simulink model "TosAeb"  (R2021a)
settings: Solver=FixedStepDiscrete  StartTime=0.0  StopTime=inf  FixedStep=0.01  RelTol=1e-3
undefined symbols (not defined in the file or in its folder): ACCModSts, AccSts1, AEB_ObjectMovingOrientation, AEB_ObjectMovingState, CN_BTCActive, CN_CurrentGearR, CN_CurrentGearR_DHT, CN_PTCActive, CN_VDCActive, CN_VSC_EspOff, CN_VSC_FCW_AEB_Error, CN_VSC_FCW_AEB_NoDispay, CN_VSC_FCW_AEB_PerfmDgdtn, CN_VSC_FCW_AEB_TempUnAvlbl, CN_VSC_VehStandstill, CNaccelAvoidMax, CNaccelAvoidMin, CNbrakePedalPress, CNcomfortStrLeftAccelMax, CNcomfortStrLeftAccelMin, CNcomfortStrRightAccelMax, CNcomfortStrRightAccelMin, CNDrvDoorOpen, CNDrvModeEco, CNDrvModeNorm, CNDrvModeOther, CNDrvModeSport, CNDrvSBRreminder, CNNull, CNobjInvalidID, CNPassDoorOpen, CNPassSBRreminder, CNPEBSAccelNegInf, CNPEBSAccelNull, CNPEBSTimeInf, CNPEBSTimeNull, CNSTOPMAX, CNSTOPNULL, CNsubjWidth, CNSwtOFF, CNSwtON, CNTTCMAX, CNTTCNULL, CNVehConfigAEBCycNone, CNVehConfigAEBNone, CNVehConfigAEBPedNone, CNVehConfigFCWNone, CNVehConfigJANone, CollisionSeverity, FALSE, FCWSnvty, JACycObjCalcInfoDefaultParams, JAPedObjCalcInfoDefaultParams, JAVehObjCalcInfoDefaultParams, latCycObjCalcInfoDefaultParams, latPedObjCalcInfoDefaultParams, longObjCalcInfoDefaultParams, ModSts, movingStatus, ObjectType ... (+1112)
libraries: simulink x3031, AEBSFunctionLib x50, Ta_Library x33, AEBSStateMachineLib x23, Mylibrary x12, simulink_extras x7, ObjSelect_lib x3, dspstat3 x2
(part 40 of 102)

== system TosAeb/FcwAebJa_Step_sys/ALG_AEBSystem/ALG_AEBController/AEB_FuncCondCalcApp/JaVehFunctionCondCalc/AEBJAVehPrefillSuppAborCond/AEBJAVehPrefillSuppCondCalc/AEBJAVehPrefillSuppObjCondCalcu ==
  note: add Active
  ObjectMovingState : Inport
  ObjectMovingOrientation : Inport  Port=2
  ObjectID : Inport  Port=3
  ObjectExistProb : Inport  Port=4
  ObjectIsObstacleProb : Inport  Port=5
  ObjectTypeCameraConfirmed : Inport  Port=6
  dy : Inport  Port=7
  vxAbsObj : Inport  Port=8
  vyRelCorr : Inport  Port=9
  ObjFusQuality : Inport  Port=10
  Compare To Constant1 : simulink/Logic and Bit Operations/Compare To Constant  relop=>  const=P_AEB_Func_JaVehPrefillObjVelSupThresMin  OutDataTypeStr=boolean
  Compare To Constant11 : simulink/Logic and Bit Operations/Compare To Constant  relop===  const=TRUE  OutDataTypeStr=boolean
  Compare To Constant14 : simulink/Logic and Bit Operations/Compare To Constant  relop===  const=AEB_ObjectMovingState.Moving  OutDataTypeStr=boolean
  Compare To Constant15 : simulink/Logic and Bit Operations/Compare To Constant  relop=~=  const=CNobjInvalidID  OutDataTypeStr=boolean
  Compare To Constant16 : simulink/Logic and Bit Operations/Compare To Constant  relop=>  const=P_AEB_Func_JaVehPrefillObjObstacleProbSupThres  OutDataTypeStr=boolean
  Compare To Constant17 : simulink/Logic and Bit Operations/Compare To Constant  relop=~=  const=CNobjInvalidID  OutDataTypeStr=boolean
  Compare To Constant18 : simulink/Logic and Bit Operations/Compare To Constant  relop===  const=TRUE  OutDataTypeStr=boolean
  Compare To Constant2 : simulink/Logic and Bit Operations/Compare To Constant  relop=<  const=P_AEB_Func_JaVehPrefillObjVelSupThresMax  OutDataTypeStr=boolean
  Compare To Constant3 : simulink/Logic and Bit Operations/Compare To Constant  relop=>  const=P_AEB_Func_JaVehPrefillObjDySupThresMin  OutDataTypeStr=boolean
  Compare To Constant4 : simulink/Logic and Bit Operations/Compare To Constant  relop===  const=AEB_ObjectMovingOrientation.InvDir  OutDataTypeStr=boolean
  Compare To Constant5 : simulink/Logic and Bit Operations/Compare To Constant  relop=<  const=P_AEB_Func_JaVehPrefillObjDySupThresMax  OutDataTypeStr=boolean
  Logical Operator{1..2,6..7} (4 alike) : Logic  AllPortsSameDT=off  OutDataTypeStr=boolean
  Logical Operator4 : Logic  Inputs=8  AllPortsSameDT=off  OutDataTypeStr=boolean
  Math Function : Math  Operator=hypot  SignedPower=on  SaturateOnIntegerOverflow=on
  Product1 : Product
  Relational Operator2 : RelationalOperator  Operator===  OutDataTypeStr=boolean  RndMeth=Simplest
  Unit Delay1 : UnitDelay  SampleTime=-1  HasFrameUpgradeWarning=on
  AEBJAVehPrefillSuppObjActiveCondFulfilled : Outport
  wires:
  Compare To Constant11/1 -> Logical Operator4/8  "ObjFusQuality"
  ObjFusQuality/1 -> Compare To Constant11/1
  Logical Operator2/1 -> Logical Operator4/6  "objVelocityCondFulfilled"
  Compare To Constant5/1 -> Logical Operator2/2
  Compare To Constant3/1 -> Logical Operator2/1
  dy/1 -> Compare To Constant5/1, Compare To Constant3/1
  Math Function/1 -> Compare To Constant1/1, Compare To Constant2/1
  Logical Operator4/1 -> AEBJAVehPrefillSuppObjActiveCondFulfilled/1
  Logical Operator1/1 -> Logical Operator4/7  "objVelocityCondFulfilled"
  Compare To Constant2/1 -> Logical Operator1/2
  Compare To Constant1/1 -> Logical Operator1/1
  vyRelCorr/1 -> Math Function/2
  vxAbsObj/1 -> Math Function/1
  Compare To Constant18/1 -> Logical Operator4/5  "objFusionCondFulfilled"
  ObjectTypeCameraConfirmed/1 -> Compare To Constant18/1
  Logical Operator7/1 -> Logical Operator4/4  "objQualityCondFulfilled"
  Compare To Constant17/1 -> Logical Operator7/1
  Compare To Constant16/1 -> Logical Operator7/2
  ObjectIsObstacleProb/1 -> Product1/2
  Product1/1 -> Compare To Constant16/1
  ObjectExistProb/1 -> Product1/1
  ObjectID/1 -> Compare To Constant15/1, Relational Operator2/1, Unit Delay1/1, Compare To Constant17/1
  Unit Delay1/1 -> Relational Operator2/2
  Relational Operator2/1 -> Logical Operator6/2
  Logical Operator6/1 -> Logical Operator4/3  "objIDCondFulfilled"
  Compare To Constant15/1 -> Logical Operator6/1
  Compare To Constant4/1 -> Logical Operator4/2  "objMovingOrientationCondFulfilled"
  ObjectMovingOrientation/1 -> Compare To Constant4/1
  Compare To Constant14/1 -> Logical Operator4/1  "objMovingStateCondFulfilled"
  ObjectMovingState/1 -> Compare To Constant14/1

== system TosAeb/FcwAebJa_Step_sys/ALG_AEBSystem/ALG_AEBController/AEB_FuncCondCalcApp/JaVehFunctionCondCalc/AEBJAVehPrefillSuppAborCond/AEBJAVehPrefillSuppCondCalc/AEBJAVehPrefillSuppSituationCondCalc ==
  initialLatDist : Inport
  restLatDist2Collision : Inport  Port=2
  tPredictTime : Inport  Port=3
  TTB : Inport  Port=4
  ObjColliFlg : Inport  Port=5
  Add : Sum  Inputs=2
  Add1 : Sum  Inputs=2
  Add2 : Sum  Inputs=2
  Compare To Constant : simulink/Logic and Bit Operations/Compare To Constant  relop===  const=TRUE  OutDataTypeStr=boolean
  Constant : Constant  Value=P_Global_VehicleWidthMin_m
  Constant1 : Constant  Value=P_AEB_Func_JaVehPrefillObjWidth
  Constant2 : Constant  Value=P_AEB_Func_JaVehPrefillBrkDistSecure
  Constant3 : Constant  Value=P_AEB_Func_JaVehAEBPrefillTtcTimeOffset
  Gain : Gain  Gain=0.5
  Gain1 : Gain  Gain=0.5
  Logical Operator : Logic  AllPortsSameDT=off  OutDataTypeStr=boolean
  Logical Operator1 : Logic  AllPortsSameDT=off  OutDataTypeStr=boolean
  Relational Operator : RelationalOperator  Operator=>  OutDataTypeStr=boolean  RndMeth=Simplest
  Relational Operator1 : RelationalOperator  Operator=<  OutDataTypeStr=boolean  RndMeth=Simplest
  Relational Operator2 : RelationalOperator  Operator=<=  OutDataTypeStr=boolean  RndMeth=Simplest
  Terminator : Terminator
  AEBJAVehPrefillActiveSituationCondFulfilled : Outport
  wires:
  restLatDist2Collision/1 -> Relational Operator2/1
  Relational Operator2/1 -> Logical Operator/2
  Relational Operator/1 -> Logical Operator/1
  Add/1 -> Add2/1
  Constant1/1 -> Gain1/1
  initialLatDist/1 -> Relational Operator/1
  Constant/1 -> Gain/1
  Gain/1 -> Add/1
  Gain1/1 -> Add/2
  Constant2/1 -> Add2/2
  Logical Operator/1 -> Terminator/1
  Add2/1 -> Relational Operator2/2, Relational Operator/2
  TTB/1 -> Add1/1
  tPredictTime/1 -> Relational Operator1/1
  Compare To Constant/1 -> Logical Operator1/2
  ObjColliFlg/1 -> Compare To Constant/1
  Add1/1 -> Relational Operator1/2
  Constant3/1 -> Add1/2
  Logical Operator1/1 -> AEBJAVehPrefillActiveSituationCondFulfilled/1
  Relational Operator1/1 -> Logical Operator1/1

== system TosAeb/FcwAebJa_Step_sys/ALG_AEBSystem/ALG_AEBController/AEB_FuncCondCalcApp/JaVehFunctionCondCalc/AEBJAVehPrefillSuppAborCond/AEBJAVehPrefillSuppCondCalc/AEBJAVehPrefillSuppSubjCondCalcu ==
  note: 2020.06.08 change or to and
  note: 2020.06.08 change and to or
  subjVel : Inport
  VehConfigIbooster : Inport  Port=2
  ESP_MasterCylindBrakePress : Inport  Port=3
  BrakePedalStatus_G : Inport  Port=4
  IB_BrkPedalAppld : Inport  Port=5
  AccrPedlPosnDiagc : Inport  Port=6
  SteerWheelAngle_Deg : Inport  Port=7
  LeftTurnSWSts : Inport  Port=8
  subjLatAccel : Inport  Port=9
  VSC_JAVehPrefillSuppCond : Inport  Port=10
  1-D Lookup Table : Lookup_n-D  NumberOfTableDimensions=1  BreakpointsForDimension1=P_AEB_Func_JaVehPrefillSubjVelStrWheelAngleMinThresX  BeginIndexSearchUsingPreviousIndexResult=on  Table=P_AEB_Func_JaVehPrefillSubjVelStrWheelAngleMinThresY  InternalRulePriority=Speed  RndMeth=Simplest  InputSameDT=on
  1-D Lookup Table1 : Lookup_n-D  NumberOfTableDimensions=1  BreakpointsForDimension1=P_AEB_Func_JaVehPrefillSubjVelStrWheelAngleMaxThresX  BeginIndexSearchUsingPreviousIndexResult=on  Table=P_AEB_Func_JaVehPrefillSubjVelStrWheelAngleMaxThresY  InternalRulePriority=Speed  RndMeth=Simplest  InputSameDT=on
  Abs : Abs
  Abs1 : Abs
  Compare To Constant2 : simulink/Logic and Bit Operations/Compare To Constant  relop===  const=FALSE  OutDataTypeStr=boolean
  Compare To Constant3 : simulink/Logic and Bit Operations/Compare To Constant  relop=<  const=P_AEB_Func_JaVehPrefillSubjLatAccelThres  OutDataTypeStr=boolean
  Compare To Constant4 : simulink/Logic and Bit Operations/Compare To Constant  relop===  const=FALSE  OutDataTypeStr=boolean
  Compare To Constant6 : simulink/Logic and Bit Operations/Compare To Constant  relop=>  const=P_AEB_Func_JaVehPrefillGasPedalSupThres  OutDataTypeStr=boolean
  Compare To Constant7 : simulink/Logic and Bit Operations/Compare To Constant  relop===  const=TRUE  OutDataTypeStr=boolean
  Compare To Constant8 : simulink/Logic and Bit Operations/Compare To Constant  relop=>=  const=P_AEB_Func_JaVehPrefillSubjVelMin  OutDataTypeStr=boolean
  Compare To Constant9 : simulink/Logic and Bit Operations/Compare To Constant  relop=<=  const=P_AEB_Func_JaVehPrefillSubjVelMax  OutDataTypeStr=boolean
  Constant : Constant  Value=P_AEB_Func_JaVehPrefillAccrCondAborDelayTime
  Constant1 : Constant  Value=Ts
  Constant2 : Constant  Value=P_AEB_Func_JaVehPrefillTurnSwtStsConfig
  Constant3 : Constant  Value=TRUE
  Logical Operator : Logic  AllPortsSameDT=off  OutDataTypeStr=boolean
  Logical Operator1 : Logic  Operator=OR  AllPortsSameDT=off  OutDataTypeStr=boolean
  Logical Operator4 : Logic  AllPortsSameDT=off  OutDataTypeStr=boolean
  Logical Operator5 : Logic  Inputs=6  AllPortsSameDT=off  OutDataTypeStr=boolean
  Relational Operator : RelationalOperator  Operator=>  OutDataTypeStr=boolean  RndMeth=Simplest
  Relational Operator1 : RelationalOperator  Operator=<  OutDataTypeStr=boolean  RndMeth=Simplest
  StateDelayAbortion : AEBSFunctionLib/StateDelayAbortion  SystemSampleTime=-1  VariantActivationTime=update diagram  Latency=0  AutoFrameSizeCalculation=off
  Switch : Switch  Criteria=u2 ~= 0
  brakeReleventSuppCond : Subsystem
  AEBJAVehPrefillVehActiveCondFulfilled : Outport
  wires:
  ESP_MasterCylindBrakePress/1 -> brakeReleventSuppCond/3(ESP_MasterCylindBrakePress)
  BrakePedalStatus_G/1 -> brakeReleventSuppCond/2(BrakePedalStatus_G)
  VehConfigIbooster/1 -> brakeReleventSuppCond/1(VehConfigIbooster)
  Compare To Constant7/1 -> Switch/1  "StrReleventActiveCondFulfilled"
  Constant2/1 -> Switch/2
  Constant3/1 -> Switch/3
  IB_BrkPedalAppld/1 -> brakeReleventSuppCond/4(IB_BrkPedalAppld)
  Compare To Constant2/1 -> Logical Operator5/2  "DrvReleventActiveCondFulfilled"
  Logical Operator1/1 -> Compare To Constant2/1
  Relational Operator1/1 -> Logical Operator4/2
  1-D Lookup Table1/1 -> Relational Operator1/2
  Compare To Constant4/1 -> Logical Operator5/6  "VSCReleventActiveCondFulfilled"
  VSC_JAVehPrefillSuppCond/1 -> Compare To Constant4/1
  Compare To Constant3/1 -> Logical Operator5/5  "LaterAccReleventActiveCondFulfilled"
  Abs1/1 -> Compare To Constant3/1
  subjLatAccel/1 -> Abs1/1
  1-D Lookup Table/1 -> Relational Operator/2
  [subjVel] -> 1-D Lookup Table/1, 1-D Lookup Table1/1
  Relational Operator/1 -> Logical Operator4/1
  Constant1/1 -> StateDelayAbortion/3
  Constant/1 -> StateDelayAbortion/2
  Compare To Constant6/1 -> StateDelayAbortion/1
  Logical Operator5/1 -> AEBJAVehPrefillVehActiveCondFulfilled/1  "AEBJAVehPrefillVehActiveCondFulfilled"
  subjVel/1 -> Compare To Constant8/1, [subjVel], Compare To Constant9/1
  Logical Operator/1 -> Logical Operator5/1  "VelocityReleventActiveCondFulfilled"
  Switch/1 -> Logical Operator5/4  "StrReleventActiveCondFulfilled"
  AccrPedlPosnDiagc/1 -> Compare To Constant6/1
  Compare To Constant9/1 -> Logical Operator/2
  brakeReleventSuppCond/1(brakeReleventSuppCond) -> Logical Operator1/1  "BrkReleventSuppCondFulfilled"
  Logical Operator4/1 -> Logical Operator5/3  "strReleventActiveCondFulfilled"
  SteerWheelAngle_Deg/1 -> Abs/1
  Abs/1 -> Relational Operator/1, Relational Operator1/1
  Compare To Constant8/1 -> Logical Operator/1
  LeftTurnSWSts/1 -> Compare To Constant7/1
  StateDelayAbortion/1 -> Logical Operator1/2  "accReleventSuppCondFulfilled"

== system TosAeb/FcwAebJa_Step_sys/ALG_AEBSystem/ALG_AEBController/AEB_FuncCondCalcApp/LongFunctionCondCalc/vehABASuppAborCondCalc/vehABAAborCondCalc/vehABAAborCondFinalJudge ==
  vehABAObjRelAborCondFulfilled : Inport
  vehABAVehRelAborCondFulfilled : Inport  Port=2
  vehABASituationRelAborCondFulfilled : Inport  Port=3
  Compare To Constant : simulink/Logic and Bit Operations/Compare To Constant  relop===  const=TRUE  OutDataTypeStr=boolean
  Compare To Constant1 : simulink/Logic and Bit Operations/Compare To Constant  relop===  const=TRUE  OutDataTypeStr=boolean
  Compare To Constant2 : simulink/Logic and Bit Operations/Compare To Constant  relop===  const=TRUE  OutDataTypeStr=boolean
  Logical Operator : Logic  Operator=OR  Inputs=3  AllPortsSameDT=off  OutDataTypeStr=boolean
  vehABAAborCondFulfilled : Outport
  wires:
  vehABAVehRelAborCondFulfilled/1 -> Compare To Constant1/1
  vehABAObjRelAborCondFulfilled/1 -> Compare To Constant/1
  vehABASituationRelAborCondFulfilled/1 -> Compare To Constant2/1
  Logical Operator/1 -> vehABAAborCondFulfilled/1
  Compare To Constant/1 -> Logical Operator/1
  Compare To Constant1/1 -> Logical Operator/3
  Compare To Constant2/1 -> Logical Operator/2

== system TosAeb/FcwAebJa_Step_sys/ALG_AEBSystem/ALG_AEBController/AEB_FuncCondCalcApp/LongFunctionCondCalc/vehABASuppAborCondCalc/vehABAAborCondCalc/vehABAObjRelAborCondCalc ==
  ObjectID : Inport
  Compare To Constant4 : simulink/Logic and Bit Operations/Compare To Constant  relop===  const=CNobjInvalidID  OutDataTypeStr=boolean
  Logical Operator1 : Logic  Operator=OR  AllPortsSameDT=off  OutDataTypeStr=boolean
  Relational Operator : RelationalOperator  Operator=~=  OutDataTypeStr=boolean  RndMeth=Simplest
  Unit Delay : UnitDelay  SampleTime=-1  HasFrameUpgradeWarning=on
  vehABAObjRelAborCondFulfilled : Outport
  wires:
  Logical Operator1/1 -> vehABAObjRelAborCondFulfilled/1  "ObjIDAborCondFulfilled"
  Compare To Constant4/1 -> Logical Operator1/1
  Relational Operator/1 -> Logical Operator1/2
  Unit Delay/1 -> Relational Operator/2
  ObjectID/1 -> Compare To Constant4/1, Relational Operator/1, Unit Delay/1

== system TosAeb/FcwAebJa_Step_sys/ALG_AEBSystem/ALG_AEBController/AEB_FuncCondCalcApp/LongFunctionCondCalc/vehABASuppAborCondCalc/vehABAAborCondCalc/vehABASituationRelAborCondCalc ==
  longCollisionSeverity : Inport
  Compare To Constant : simulink/Logic and Bit Operations/Compare To Constant  relop=~=  const=CollisionSeverity.Low  OutDataTypeStr=boolean
  Compare To Constant1 : simulink/Logic and Bit Operations/Compare To Constant  relop=~=  const=CollisionSeverity.Medium  OutDataTypeStr=boolean
  Compare To Constant2 : simulink/Logic and Bit Operations/Compare To Constant  relop=~=  const=CollisionSeverity.High  OutDataTypeStr=boolean
  Logical Operator : Logic  Inputs=3  AllPortsSameDT=off  OutDataTypeStr=boolean
  vehABASituationRelAborCondFulfilled : Outport
  wires:
  Logical Operator/1 -> vehABASituationRelAborCondFulfilled/1
  Compare To Constant/1 -> Logical Operator/1
  longCollisionSeverity/1 -> Compare To Constant/1, Compare To Constant2/1, Compare To Constant1/1
  Compare To Constant2/1 -> Logical Operator/3
  Compare To Constant1/1 -> Logical Operator/2

== system TosAeb/FcwAebJa_Step_sys/ALG_AEBSystem/ALG_AEBController/AEB_FuncCondCalcApp/LongFunctionCondCalc/vehABASuppAborCondCalc/vehABAAborCondCalc/vehABAVehRelAborCondCalc ==
  SteerWheelAngle : Inport
  SteerWheelSpd : Inport  Port=2
  AccrPedlPosnDiagc : Inport  Port=3
  vehStateCalcABAAborFlg : Inport  Port=4
  DriverAborCondJudge : Subsystem
  vehPreWarningVehRelActiveCondJudge : Subsystem
  vehABAVehRelAborCondFulfilled : Outport
  wires:
  AccrPedlPosnDiagc/1 -> DriverAborCondJudge/3(AccrPedlPosnDiagc)
  SteerWheelSpd/1 -> DriverAborCondJudge/2(SteerWheelSpd)
  SteerWheelAngle/1 -> DriverAborCondJudge/1(SteerWheelAngle)
  vehPreWarningVehRelActiveCondJudge/1(vehABAVehRelAborCondFulfilled) -> vehABAVehRelAborCondFulfilled/1
  vehStateCalcABAAborFlg/1 -> vehPreWarningVehRelActiveCondJudge/2(vehStateCalcABAAborFlg)
  DriverAborCondJudge/1(driverAborCondFlg) -> vehPreWarningVehRelActiveCondJudge/1(driverAborCondFlg)

== system TosAeb/FcwAebJa_Step_sys/ALG_AEBSystem/ALG_AEBController/AEB_FuncCondCalcApp/LongFunctionCondCalc/vehABASuppAborCondCalc/vehABASuppCondCalc/vehABAObjRelActiveCondCalc ==
  ObjectMovingState : Inport
  ObjectTypeCameraConfirmed : Inport  Port=2
  AEB_PedResp : Inport  Port=3
  ObjectType : Inport  Port=4
  subjCurvTraj : Inport  Port=5
  subjLatAccel : Inport  Port=6
  subjVel : Inport  Port=7
  vehConfigAEBCyc : Inport  Port=8
  vehConfigAEBPed : Inport  Port=9
  ObjectMovingOrientation : Inport  Port=10
  ObjectID : Inport  Port=11
  ObjectExistProb : Inport  Port=12
  ObjectIsObstacleProb : Inport  Port=13
  ObjFusQuality : Inport  Port=14
  Compare To Constant : simulink/Logic and Bit Operations/Compare To Constant  relop===  const=AEB_ObjectMovingState.Still  OutDataTypeStr=boolean
  Compare To Constant1 : simulink/Logic and Bit Operations/Compare To Constant  relop===  const=AEB_ObjectMovingState.Moving  OutDataTypeStr=boolean
  Compare To Constant10 : simulink/Logic and Bit Operations/Compare To Constant  relop===  const=TRUE  OutDataTypeStr=boolean
  Compare To Constant12 : simulink/Logic and Bit Operations/Compare To Constant  relop===  const=FALSE  OutDataTypeStr=boolean
  Compare To Constant2 : simulink/Logic and Bit Operations/Compare To Constant  relop===  const=AEB_ObjectMovingState.Stopped  OutDataTypeStr=boolean
  Compare To Constant3 : simulink/Logic and Bit Operations/Compare To Constant  relop===  const=AEB_ObjectMovingOrientation.SameDir  OutDataTypeStr=boolean
  Compare To Constant4 : simulink/Logic and Bit Operations/Compare To Constant  relop=~=  const=CNobjInvalidID  OutDataTypeStr=boolean
  Compare To Constant5 : simulink/Logic and Bit Operations/Compare To Constant  relop=>  const=P_AEB_Func_vehABAObjObstacleProbActivationThres  OutDataTypeStr=boolean
  Compare To Constant6 : simulink/Logic and Bit Operations/Compare To Constant  relop=~=  const=CNobjInvalidID  OutDataTypeStr=boolean
  Compare To Constant8 : simulink/Logic and Bit Operations/Compare To Constant  relop===  const=TRUE  OutDataTypeStr=boolean
  Constant3 : Constant  Value=P_AEB_Func_vehABASubjVelActivationThresForPedCyc
  Constant4 : Constant  Value=P_AEB_Func_vehABASubjCurvTrajActivationThresForPedCyc
  Constant5 : Constant  Value=P_AEB_Func_vehABASubjLatAccelActivationThresForPedCyc
  Logical Operator : Logic  Operator=OR  Inputs=3  AllPortsSameDT=off  OutDataTypeStr=boolean
  Logical Operator1 : Logic  AllPortsSameDT=off  OutDataTypeStr=boolean
  Logical Operator2 : Logic  AllPortsSameDT=off  OutDataTypeStr=boolean
  Logical Operator5 : Logic  Inputs=7  AllPortsSameDT=off  OutDataTypeStr=boolean
  PedCycObjCondSuppJudgeFlow : AEBSFunctionLib/PedCycObjCondSuppJudgeFlow  SystemSampleTime=-1  VariantActivationTime=update diagram  Latency=0  AutoFrameSizeCalculation=off
  Product : Product
  Relational Operator : RelationalOperator  Operator===  OutDataTypeStr=boolean  RndMeth=Simplest
  Unit Delay : UnitDelay  SampleTime=-1  HasFrameUpgradeWarning=on
  vehABAObjRelActiveCondFulfilled : Outport
  wires:
  Compare To Constant10/1 -> Logical Operator5/7  "ObjFusQuality"
  ObjFusQuality/1 -> Compare To Constant10/1
  Compare To Constant8/1 -> Logical Operator5/2  "ObjFusionInfoConfirm"
  Constant5/1 -> PedCycObjCondSuppJudgeFlow/11
  Constant4/1 -> PedCycObjCondSuppJudgeFlow/10
  Constant3/1 -> PedCycObjCondSuppJudgeFlow/9
  AEB_PedResp/1 -> PedCycObjCondSuppJudgeFlow/8
  vehConfigAEBPed/1 -> PedCycObjCondSuppJudgeFlow/7
  vehConfigAEBCyc/1 -> PedCycObjCondSuppJudgeFlow/6
  subjVel/1 -> PedCycObjCondSuppJudgeFlow/5
  subjLatAccel/1 -> PedCycObjCondSuppJudgeFlow/4
  subjCurvTraj/1 -> PedCycObjCondSuppJudgeFlow/3
  ObjectType/1 -> PedCycObjCondSuppJudgeFlow/2
  ObjectTypeCameraConfirmed/1 -> Compare To Constant8/1, PedCycObjCondSuppJudgeFlow/1
  Compare To Constant/1 -> Logical Operator/3
  Compare To Constant12/1 -> Logical Operator5/3  "pedCycReleventCondFulfilled"
  ObjectMovingState/1 -> Compare To Constant/1, Compare To Constant2/1, Compare To Constant1/1
  Compare To Constant2/1 -> Logical Operator/2
  Compare To Constant1/1 -> Logical Operator/1
  ObjectMovingOrientation/1 -> Compare To Constant3/1
  ObjectID/1 -> Compare To Constant6/1, Unit Delay/1, Relational Operator/1, Compare To Constant4/1
  Unit Delay/1 -> Relational Operator/2
  ObjectExistProb/1 -> Product/1
  ObjectIsObstacleProb/1 -> Product/2
  Compare To Constant6/1 -> Logical Operator2/1
  Compare To Constant5/1 -> Logical Operator2/2
  Product/1 -> Compare To Constant5/1
  Relational Operator/1 -> Logical Operator1/2
  Compare To Constant4/1 -> Logical Operator1/1
  Logical Operator5/1 -> vehABAObjRelActiveCondFulfilled/1
  Compare To Constant3/1 -> Logical Operator5/4  "objMovingOrientationCondFulfilled"
  Logical Operator2/1 -> Logical Operator5/6  "objQualityCondFulfilled"
  PedCycObjCondSuppJudgeFlow/1 -> Compare To Constant12/1  "pedCycObjCondSuppFlg"
  Logical Operator/1 -> Logical Operator5/1  "objMovingStateCondFulfilled"
  Logical Operator1/1 -> Logical Operator5/5  "objIDCondFulfilled"

== system TosAeb/FcwAebJa_Step_sys/ALG_AEBSystem/ALG_AEBController/AEB_FuncCondCalcApp/LongFunctionCondCalc/vehABASuppAborCondCalc/vehABASuppCondCalc/vehABASituationRelActiveCondCalc ==
  longCollisionSeverity : Inport
  overlapRatio : Inport  Port=2
  dxFrontBumper : Inport  Port=3
  probEscape : Inport  Port=4
  Compare To Constant : simulink/Logic and Bit Operations/Compare To Constant  relop===  const=CollisionSeverity.Low  OutDataTypeStr=boolean
  Compare To Constant1 : simulink/Logic and Bit Operations/Compare To Constant  relop===  const=CollisionSeverity.Medium  OutDataTypeStr=boolean
  Compare To Constant2 : simulink/Logic and Bit Operations/Compare To Constant  relop===  const=CollisionSeverity.High  OutDataTypeStr=boolean
  Compare To Constant3 : simulink/Logic and Bit Operations/Compare To Constant  relop=>  const=P_AEB_Func_vehABAEscapeActivationThres  OutDataTypeStr=boolean
  Compare To Constant5 : simulink/Logic and Bit Operations/Compare To Constant  relop===  const=FALSE  OutDataTypeStr=boolean
  Logical Operator : Logic  Operator=OR  Inputs=3  AllPortsSameDT=off  OutDataTypeStr=boolean
  Logical Operator1 : Logic  Inputs=3  AllPortsSameDT=off  OutDataTypeStr=boolean
  OverlapThesLookupTable : Lookup_n-D  NumberOfTableDimensions=1  BreakpointsForDimension1=P_AEB_Func_vehABAOverlapActivationThresX  BeginIndexSearchUsingPreviousIndexResult=on  Table=P_AEB_Func_vehABAOverlapActivationThresY  ExtrapMethod=Clip  InternalRulePriority=Speed  RndMeth=Simplest  InputSameDT=on
  Relational Operator : RelationalOperator  Operator=>  OutDataTypeStr=boolean  RndMeth=Simplest
  vehABASituationRelActiveCondFulfilled : Outport
  wires:
  Logical Operator1/1 -> vehABASituationRelActiveCondFulfilled/1
  Compare To Constant2/1 -> Logical Operator/3
  Compare To Constant/1 -> Logical Operator/1
  overlapRatio/1 -> Relational Operator/1
  Compare To Constant1/1 -> Logical Operator/2
  probEscape/1 -> Compare To Constant3/1
  longCollisionSeverity/1 -> Compare To Constant/1, Compare To Constant1/1, Compare To Constant2/1
  Compare To Constant3/1 -> Compare To Constant5/1  "probEscapeFulfilled"
  dxFrontBumper/1 -> OverlapThesLookupTable/1
  OverlapThesLookupTable/1 -> Relational Operator/2
  Relational Operator/1 -> Logical Operator1/2  "OverlapCondFulfilled"
  Logical Operator/1 -> Logical Operator1/1  "collisionLvlCondFulfilled"
  Compare To Constant5/1 -> Logical Operator1/3

== system TosAeb/FcwAebJa_Step_sys/ALG_AEBSystem/ALG_AEBController/AEB_FuncCondCalcApp/LongFunctionCondCalc/vehABASuppAborCondCalc/vehABASuppCondCalc/vehABASuppCondFinalJudge ==
  vehABAObjRelActiveCondFulfilled : Inport
  vehABAVehRelActiveCondFulfilled : Inport  Port=2
  vehABASituationRelActiveCondFulfilled : Inport  Port=3
  Compare To Constant : simulink/Logic and Bit Operations/Compare To Constant  relop===  const=FALSE  OutDataTypeStr=boolean
  Compare To Constant1 : simulink/Logic and Bit Operations/Compare To Constant  relop===  const=FALSE  OutDataTypeStr=boolean
  Compare To Constant2 : simulink/Logic and Bit Operations/Compare To Constant  relop===  const=FALSE  OutDataTypeStr=boolean
  Logical Operator : Logic  Operator=OR  Inputs=3  AllPortsSameDT=off  OutDataTypeStr=boolean
  vehABASuppCondFulfilled : Outport
  wires:
  vehABAVehRelActiveCondFulfilled/1 -> Compare To Constant1/1
  vehABAObjRelActiveCondFulfilled/1 -> Compare To Constant/1
  vehABASituationRelActiveCondFulfilled/1 -> Compare To Constant2/1
  Logical Operator/1 -> vehABASuppCondFulfilled/1
  Compare To Constant/1 -> Logical Operator/1
  Compare To Constant1/1 -> Logical Operator/2
  Compare To Constant2/1 -> Logical Operator/3

== system TosAeb/FcwAebJa_Step_sys/ALG_AEBSystem/ALG_AEBController/AEB_FuncCondCalcApp/LongFunctionCondCalc/vehABASuppAborCondCalc/vehABASuppCondCalc/vehABAVehRelActiveCondCalc ==
  subjVel : Inport
  ObjectMovingState : Inport  Port=2
  vehStateCalcABASuppFlg : Inport  Port=3
  SteerWheelAngle : Inport  Port=4
  SteerWheelSpd : Inport  Port=5
  AccrPedlPosnDiagc : Inport  Port=6
  DriverCondJudge : Subsystem
  subjVelWork : Subsystem
  vehABAVehRelActiveCondJudge : Subsystem
  vehABAVehRelActiveCondFulfilled : Outport
  wires:
  AccrPedlPosnDiagc/1 -> DriverCondJudge/3(AccrPedlPosnDiagc)
  SteerWheelSpd/1 -> DriverCondJudge/2(SteerWheelSpd)
  SteerWheelAngle/1 -> DriverCondJudge/1(SteerWheelAngle)
  subjVelWork/2(subjVelWorkMin) -> vehABAVehRelActiveCondJudge/3(subjVelWorkMin)
  subjVelWork/1(subjVelWorkMax) -> vehABAVehRelActiveCondJudge/2(subjVelWorkMax)
  ObjectMovingState/1 -> subjVelWork/1(ObjectMovingState)
  DriverCondJudge/1(driverSuppCondFlg) -> vehABAVehRelActiveCondJudge/4(driverSuppCondFlg)
  vehStateCalcABASuppFlg/1 -> vehABAVehRelActiveCondJudge/5(vehStateCalcABASuppFlg)
  vehABAVehRelActiveCondJudge/1(vehABAVehRelActiveCondFulfilled) -> vehABAVehRelActiveCondFulfilled/1
  subjVel/1 -> vehABAVehRelActiveCondJudge/1(subjVel)

== system TosAeb/FcwAebJa_Step_sys/ALG_AEBSystem/ALG_AEBController/AEB_FuncCondCalcApp/LongFunctionCondCalc/vehAEBfullSuppAborCondCalc/ vehAEBfullSuppCondCalc/vehAEBfullObjRelActiveCondCalc ==
  ObjectMovingState : Inport
  dz : Inport  Port=2
  dxFrontBumper : Inport  Port=3
  ObjectTypeCameraConfirmed : Inport  Port=4
  ObjectType : Inport  Port=5
  subjCurvTraj : Inport  Port=6
  subjLatAccel : Inport  Port=7
  subjVel : Inport  Port=8
  vehConfigAEBCyc : Inport  Port=9
  vehConfigAEBPed : Inport  Port=10
  AEB_PedResp : Inport  Port=11
  ObjectMovingOrientation : Inport  Port=12
  ObjectID : Inport  Port=13
  ObjectExistProb : Inport  Port=14
  ObjectIsObstacleProb : Inport  Port=15
  dxVariance : Inport  Port=16
  ObjFusQuality : Inport  Port=17
  Compare To Constant : simulink/Logic and Bit Operations/Compare To Constant  relop===  const=AEB_ObjectMovingState.Still  OutDataTypeStr=boolean
  Compare To Constant1 : simulink/Logic and Bit Operations/Compare To Constant  relop===  const=AEB_ObjectMovingState.Moving  OutDataTypeStr=boolean
  Compare To Constant10 : simulink/Logic and Bit Operations/Compare To Constant  relop=<  const=P_AEB_Func_vehAEBfullObjHeightThres  OutDataTypeStr=boolean
  Compare To Constant11 : simulink/Logic and Bit Operations/Compare To Constant  relop===  const=TRUE  OutDataTypeStr=boolean
  Compare To Constant12 : simulink/Logic and Bit Operations/Compare To Constant  relop===  const=FALSE  OutDataTypeStr=boolean
  Compare To Constant13 : simulink/Logic and Bit Operations/Compare To Constant  relop===  const=AEB_ObjectMovingState.Still  OutDataTypeStr=boolean
  Compare To Constant14 : simulink/Logic and Bit Operations/Compare To Constant  relop=>  const=P_AEB_Func_vehAEBfullDxActivationSubjVelThresSO  OutDataTypeStr=boolean
  Compare To Constant15 : simulink/Logic and Bit Operations/Compare To Constant  relop===  const=TRUE  OutDataTypeStr=boolean
  Compare To Constant2 : simulink/Logic and Bit Operations/Compare To Constant  relop===  const=AEB_ObjectMovingState.Stopped  OutDataTypeStr=boolean
  Compare To Constant3 : simulink/Logic and Bit Operations/Compare To Constant  relop===  const=AEB_ObjectMovingOrientation.SameDir  OutDataTypeStr=boolean
  Compare To Constant4 : simulink/Logic and Bit Operations/Compare To Constant  relop=~=  const=CNobjInvalidID  OutDataTypeStr=boolean
  Compare To Constant5 : simulink/Logic and Bit Operations/Compare To Constant  relop=>  const=P_AEB_Func_vehAEBfullObjObstacleProbActivationThres  OutDataTypeStr=boolean
  Compare To Constant6 : simulink/Logic and Bit Operations/Compare To Constant  relop=~=  const=CNobjInvalidID  OutDataTypeStr=boolean
  Compare To Constant7 : simulink/Logic and Bit Operations/Compare To Constant  relop===  const=AEB_ObjectMovingState.Moving  OutDataTypeStr=boolean
  Compare To Constant8 : simulink/Logic and Bit Operations/Compare To Constant  relop===  const=AEB_ObjectMovingState.Stopped  OutDataTypeStr=boolean
  Compare To Constant9 : simulink/Logic and Bit Operations/Compare To Constant  relop=<  const=P_AEB_Func_vehAEBfullDxVarActivationThres  OutDataTypeStr=boolean
  Constant : Constant  Value=P_AEB_Func_vehAEBfullDxActivationThresSOHighSpd
  Constant1 : Constant  Value=P_AEB_Func_vehAEBfullDxActivationThresMO
  Constant2 : Constant  Value=TRUE
  Constant3 : Constant  Value=P_AEB_Func_vehAEBfullSubjVelActivationThresForPedCyc
  Constant4 : Constant  Value=P_AEB_Func_vehAEBfullSubjCurvTrajActivationThresForPedCyc
  Constant5 : Constant  Value=P_AEB_Func_vehAEBfullObjSubjLatAccelActivationThresForPedCyc
  Constant7 : Constant  Value=P_AEB_Func_vehAEBfullDxActivationThresSOLowSpd
  Logical Operator : Logic  Operator=OR  Inputs=3  AllPortsSameDT=off  OutDataTypeStr=boolean
  Logical Operator1 : Logic  AllPortsSameDT=off  OutDataTypeStr=boolean
  Logical Operator2 : Logic  AllPortsSameDT=off  OutDataTypeStr=boolean
  Logical Operator3 : Logic  Operator=OR  AllPortsSameDT=off  OutDataTypeStr=boolean
  Logical Operator5 : Logic  Inputs=10  AllPortsSameDT=off  OutDataTypeStr=boolean
  PedCycObjCondSuppJudgeFlow : AEBSFunctionLib/PedCycObjCondSuppJudgeFlow  SystemSampleTime=-1  VariantActivationTime=update diagram  Latency=0  AutoFrameSizeCalculation=off
  Product : Product
  Relational Operator : RelationalOperator  Operator===  OutDataTypeStr=boolean  RndMeth=Simplest
  Relational Operator1 : RelationalOperator  Operator=<=  OutDataTypeStr=boolean  RndMeth=Simplest
  Switch : Switch  Criteria=u2 ~= 0
  Switch1 : Switch  Criteria=u2 ~= 0
  Switch2 : Switch  Criteria=u2 ~= 0
  Unit Delay : UnitDelay  SampleTime=-1  HasFrameUpgradeWarning=on
  vehAEBfullObjRelActiveCondFulfilled : Outport
  wires:
  Compare To Constant15/1 -> Logical Operator5/10  "ObjFusQuality"
  ObjFusQuality/1 -> Compare To Constant15/1
  Switch2/1 -> Switch/3
  Constant7/1 -> Switch2/3
  Constant/1 -> Switch2/1
  Compare To Constant14/1 -> Switch2/2
  [subjVel] -> Compare To Constant14/1
  Compare To Constant11/1 -> Logical Operator5/4  "ObjFusionCameraConfirm"
  Compare To Constant13/1 -> Switch1/2
  AEB_PedResp/1 -> PedCycObjCondSuppJudgeFlow/8
  vehConfigAEBPed/1 -> PedCycObjCondSuppJudgeFlow/7
  vehConfigAEBCyc/1 -> PedCycObjCondSuppJudgeFlow/6
  subjCurvTraj/1 -> PedCycObjCondSuppJudgeFlow/3
  subjVel/1 -> PedCycObjCondSuppJudgeFlow/5, [subjVel]
  subjLatAccel/1 -> PedCycObjCondSuppJudgeFlow/4
  ObjectType/1 -> PedCycObjCondSuppJudgeFlow/2
  ObjectTypeCameraConfirmed/1 -> PedCycObjCondSuppJudgeFlow/1, Compare To Constant11/1
  Constant5/1 -> PedCycObjCondSuppJudgeFlow/11
  Constant4/1 -> PedCycObjCondSuppJudgeFlow/10
  Constant3/1 -> PedCycObjCondSuppJudgeFlow/9
  Constant2/1 -> Switch1/3
  Compare To Constant10/1 -> Switch1/1
  Switch1/1 -> Logical Operator5/2  "objHeightInfoFulfilled"
  Compare To Constant/1 -> Logical Operator/3
  dz/1 -> Compare To Constant10/1
  Switch/1 -> Relational Operator1/2
  Constant1/1 -> Switch/1
  Logical Operator3/1 -> Switch/2
  Compare To Constant7/1 -> Logical Operator3/2
  Compare To Constant8/1 -> Logical Operator3/1
  Compare To Constant12/1 -> Logical Operator5/5  "pedCycReleventCondFulfilled"
  ObjectMovingState/1 -> Compare To Constant1/1, Compare To Constant2/1, Compare To Constant/1, Compare To Constant13/1, Compare To Constant7/1, Compare To Constant8/1
  Compare To Constant2/1 -> Logical Operator/2
  Compare To Constant1/1 -> Logical Operator/1
  ObjectMovingOrientation/1 -> Compare To Constant3/1
  ObjectID/1 -> Compare To Constant6/1, Unit Delay/1, Relational Operator/1, Compare To Constant4/1
  Unit Delay/1 -> Relational Operator/2
  ObjectExistProb/1 -> Product/1
  ObjectIsObstacleProb/1 -> Product/2
  Compare To Constant6/1 -> Logical Operator2/1
  Compare To Constant5/1 -> Logical Operator2/2
  Product/1 -> Compare To Constant5/1
  dxFrontBumper/1 -> Relational Operator1/1
  dxVariance/1 -> Compare To Constant9/1
  Relational Operator/1 -> Logical Operator1/2
  Compare To Constant4/1 -> Logical Operator1/1
  Logical Operator5/1 -> vehAEBfullObjRelActiveCondFulfilled/1  "vehAEBfullObjRelActiveCondFulfilled"
  Compare To Constant3/1 -> Logical Operator5/6  "objMovingOrientationCondFulfilled"
  Logical Operator2/1 -> Logical Operator5/8  "objQualityCondFulfilled"
  PedCycObjCondSuppJudgeFlow/1 -> Compare To Constant12/1  "pedCycObjCondSuppFlg"
  Relational Operator1/1 -> Logical Operator5/3  "dxRelCondFulfilled"
  Logical Operator/1 -> Logical Operator5/1  "objMovingStateCondFulfilled"
  Logical Operator1/1 -> Logical Operator5/7  "objIDCondFulfilled"
  Compare To Constant9/1 -> Logical Operator5/9  "dxRelVarCondFulfilled"

== system TosAeb/FcwAebJa_Step_sys/ALG_AEBSystem/ALG_AEBController/AEB_FuncCondCalcApp/LongFunctionCondCalc/vehAEBfullSuppAborCondCalc/ vehAEBfullSuppCondCalc/vehAEBfullSituationRelActiveCondCalc ==
  ObjectTypeCameraConfirmed : Inport
  dxFrontBumper : Inport  Port=2
  longCollisionSeverity : Inport  Port=3
  overlapRatio : Inport  Port=4
  LCK_Active : Inport  Port=5
  probEscape : Inport  Port=6
  subjCurvTraj : Inport  Port=7
  overlapThesCalc : Subsystem
  vehAEBfullSituationRelActiveCondCalc : Subsystem
  vehAEBfullSituationRelActiveCondFulfilled : Outport
  wires:
  LCK_Active/1 -> vehAEBfullSituationRelActiveCondCalc/4(LCK_Active)
  vehAEBfullSituationRelActiveCondCalc/1(vehAEBfullSituationRelActiveCondFulfilled) -> vehAEBfullSituationRelActiveCondFulfilled/1  "vehAEBfullSituationRelActiveCondFulfilled"
  subjCurvTraj/1 -> vehAEBfullSituationRelActiveCondCalc/6(subjCurvTraj)
  probEscape/1 -> vehAEBfullSituationRelActiveCondCalc/5(probEscape)
  overlapThesCalc/1(overlapThes) -> vehAEBfullSituationRelActiveCondCalc/1(overlapThes)
  overlapRatio/1 -> vehAEBfullSituationRelActiveCondCalc/3(overlapRatio)
  longCollisionSeverity/1 -> vehAEBfullSituationRelActiveCondCalc/2(longCollisionSeverity)
  dxFrontBumper/1 -> overlapThesCalc/2(dxFrontBumper)
  ObjectTypeCameraConfirmed/1 -> overlapThesCalc/1(ObjectTypeCameraConfirmed)

== system TosAeb/FcwAebJa_Step_sys/ALG_AEBSystem/ALG_AEBController/AEB_FuncCondCalcApp/LongFunctionCondCalc/vehAEBfullSuppAborCondCalc/ vehAEBfullSuppCondCalc/vehAEBfullSuppCondFinalJudge ==
  vehAEBfullObjRelActiveCondFulfilled : Inport
  vehAEBfullVehRelActiveCondFulfilled : Inport  Port=2
  vehAEBfullSituationRelActiveCondFulfilled : Inport  Port=3
  Compare To Constant : simulink/Logic and Bit Operations/Compare To Constant  relop===  const=FALSE  OutDataTypeStr=boolean
  Compare To Constant1 : simulink/Logic and Bit Operations/Compare To Constant  relop===  const=FALSE  OutDataTypeStr=boolean
  Compare To Constant2 : simulink/Logic and Bit Operations/Compare To Constant  relop===  const=FALSE  OutDataTypeStr=boolean
  Logical Operator : Logic  Operator=OR  Inputs=3  AllPortsSameDT=off  OutDataTypeStr=boolean
  vehAEBfullSuppCondFulfilled : Outport
  wires:
  vehAEBfullVehRelActiveCondFulfilled/1 -> Compare To Constant1/1
  vehAEBfullObjRelActiveCondFulfilled/1 -> Compare To Constant/1
  vehAEBfullSituationRelActiveCondFulfilled/1 -> Compare To Constant2/1
  Logical Operator/1 -> vehAEBfullSuppCondFulfilled/1
  Compare To Constant/1 -> Logical Operator/1
  Compare To Constant1/1 -> Logical Operator/2
  Compare To Constant2/1 -> Logical Operator/3

== system TosAeb/FcwAebJa_Step_sys/ALG_AEBSystem/ALG_AEBController/AEB_FuncCondCalcApp/LongFunctionCondCalc/vehAEBfullSuppAborCondCalc/ vehAEBfullSuppCondCalc/vehAEBfullVehRelActiveCondCalc ==
  ObjectTypeCameraConfirmed : Inport
  ObjectMovingState : Inport  Port=2
  vehStateCalcAEBfullSuppFlg : Inport  Port=3
  SteerWheelAngle : Inport  Port=4
  SteerWheelSpd : Inport  Port=5
  VehConfigIbooster : Inport  Port=6
  ESPmasterCylindBrakePress : Inport  Port=7
  BrakePedalStatus_G : Inport  Port=8
  subjLongAccel : Inport  Port=9
  subjVel : Inport  Port=10
  AccrPedlPosnDiagc : Inport  Port=11
  DriverCondJudge : Subsystem
  subjVelWork : Subsystem
  vehAEBfullVehRelActiveCondJudge : Subsystem
  vehAEBfullVehRelActiveCondFulfilled : Outport
  wires:
  VehConfigIbooster/1 -> DriverCondJudge/3(VehConfigIbooster)
  [subVel] -> vehAEBfullVehRelActiveCondJudge/1(subjVel)
  AccrPedlPosnDiagc/1 -> DriverCondJudge/8(AccrPedlPosnDiagc)
  subjVel/1 -> DriverCondJudge/7(subjVel), [subVel]
  subjLongAccel/1 -> DriverCondJudge/6(subjLongAccel)
  BrakePedalStatus_G/1 -> DriverCondJudge/5(BrakePedalStatus_G)
  ESPmasterCylindBrakePress/1 -> DriverCondJudge/4(ESPmasterCylindBrakePress)
  SteerWheelSpd/1 -> DriverCondJudge/2(SteerWheelSpd)
  SteerWheelAngle/1 -> DriverCondJudge/1(SteerWheelAngle)
  subjVelWork/2(subjVelWorkMin) -> vehAEBfullVehRelActiveCondJudge/3(subjVelWorkMin)
  subjVelWork/1(subjVelWorkMax) -> vehAEBfullVehRelActiveCondJudge/2(subjVelWorkMax)
  ObjectMovingState/1 -> subjVelWork/2(ObjectMovingState)
  ObjectTypeCameraConfirmed/1 -> subjVelWork/1(ObjectTypeCameraConfirmed)
  DriverCondJudge/1(driverSuppCondFlg) -> vehAEBfullVehRelActiveCondJudge/4(driverSuppCondFlg)
  vehStateCalcAEBfullSuppFlg/1 -> vehAEBfullVehRelActiveCondJudge/5(vehStateCalcAEBfullSuppFlg)
  vehAEBfullVehRelActiveCondJudge/1(vehAEBfullVehRelActiveCondFulfilled) -> vehAEBfullVehRelActiveCondFulfilled/1  "vehAEBfullVehRelActiveCondFulfilled"

== system TosAeb/FcwAebJa_Step_sys/ALG_AEBSystem/ALG_AEBController/AEB_FuncCondCalcApp/LongFunctionCondCalc/vehAEBfullSuppAborCondCalc/vehAEBfullAborCondCalc/vehAEBfullAborCondFinalJudge ==
  note: vehAEBfullAborCondStandstillFulfilled
  vehAEBfullObjRelAborCondFulfilled : Inport
  vehAEBfullVehRelAborCondFulfilled : Inport  Port=2
  vehAEBfullSituationRelAborCondFulfilled : Inport  Port=3
  Compare To Constant : simulink/Logic and Bit Operations/Compare To Constant  relop===  const=TRUE  OutDataTypeStr=boolean
  Compare To Constant1 : simulink/Logic and Bit Operations/Compare To Constant  relop===  const=TRUE  OutDataTypeStr=boolean
  Compare To Constant2 : simulink/Logic and Bit Operations/Compare To Constant  relop===  const=TRUE  OutDataTypeStr=boolean
  Logical Operator : Logic  Operator=OR  Inputs=3  AllPortsSameDT=off  OutDataTypeStr=boolean
  vehAEBfullAborCondNoStandstillFulfilled : Outport
  vehAEBfullAborCondStandstillFulfilled : Outport  Port=2
  wires:
  vehAEBfullVehRelAborCondFulfilled/1 -> Compare To Constant1/1
  vehAEBfullObjRelAborCondFulfilled/1 -> Compare To Constant/1
  vehAEBfullSituationRelAborCondFulfilled/1 -> Compare To Constant2/1
  Logical Operator/1 -> vehAEBfullAborCondNoStandstillFulfilled/1  "vehAEBfullAborCondNoStandstillFulfilled"
  Compare To Constant/1 -> Logical Operator/1
  Compare To Constant1/1 -> vehAEBfullAborCondStandstillFulfilled/1, Logical Operator/3
  Compare To Constant2/1 -> Logical Operator/2

== system TosAeb/FcwAebJa_Step_sys/ALG_AEBSystem/ALG_AEBController/AEB_FuncCondCalcApp/LongFunctionCondCalc/vehAEBfullSuppAborCondCalc/vehAEBfullAborCondCalc/vehAEBfullObjRelAborCondCalc ==
  ObjectID : Inport
  ObjectExistProb : Inport  Port=2
  ObjectIsObstacleProb : Inport  Port=3
  dxVariance : Inport  Port=4
  Compare To Constant1 : simulink/Logic and Bit Operations/Compare To Constant  relop=>  const=P_AEB_Func_vehAEBfullDxVarAborThres  OutDataTypeStr=boolean
  Compare To Constant4 : simulink/Logic and Bit Operations/Compare To Constant  relop===  const=CNobjInvalidID  OutDataTypeStr=boolean
  Compare To Constant6 : simulink/Logic and Bit Operations/Compare To Constant  relop=<  const=P_AEB_Func_vehAEBfullObjObstacleProbAborThres  OutDataTypeStr=boolean
  Compare To Constant7 : simulink/Logic and Bit Operations/Compare To Constant  relop=~=  const=CNobjInvalidID  OutDataTypeStr=boolean
  Logical Operator1 : Logic  Operator=OR  AllPortsSameDT=off  OutDataTypeStr=boolean
  Logical Operator3 : Logic  AllPortsSameDT=off  OutDataTypeStr=boolean
  Logical Operator5 : Logic  Operator=OR  Inputs=3  AllPortsSameDT=off  OutDataTypeStr=boolean
  Product : Product
  Relational Operator : RelationalOperator  Operator=~=  OutDataTypeStr=boolean  RndMeth=Simplest
  Unit Delay : UnitDelay  SampleTime=-1  HasFrameUpgradeWarning=on
  vehAEBfullObjRelAborCondFulfilled : Outport
  wires:
  Compare To Constant1/1 -> Logical Operator5/3  "dxRelVarAborCondFulfilled"
  dxVariance/1 -> Compare To Constant1/1
  Logical Operator1/1 -> Logical Operator5/1  "ObjIDAborCondFulfilled"
  Compare To Constant4/1 -> Logical Operator1/1
  Relational Operator/1 -> Logical Operator1/2
  Unit Delay/1 -> Relational Operator/2
  ObjectID/1 -> Compare To Constant4/1, Relational Operator/1, Compare To Constant7/1, Unit Delay/1
  Compare To Constant6/1 -> Logical Operator3/2
  Logical Operator3/1 -> Logical Operator5/2  "objQualityAborCondFulfilled"
  ObjectIsObstacleProb/1 -> Product/2
  Compare To Constant7/1 -> Logical Operator3/1
  Product/1 -> Compare To Constant6/1
  Logical Operator5/1 -> vehAEBfullObjRelAborCondFulfilled/1  "vehAEBfullObjRelAborCondFulfilled"
  ObjectExistProb/1 -> Product/1

== system TosAeb/FcwAebJa_Step_sys/ALG_AEBSystem/ALG_AEBController/AEB_FuncCondCalcApp/LongFunctionCondCalc/vehAEBfullSuppAborCondCalc/vehAEBfullAborCondCalc/vehAEBfullSituationRelAborCondCalc ==
  overlapRatio : Inport
  dxFrontBumper : Inport  Port=2
  probEscape : Inport  Port=3
  ObjectMovingState : Inport  Port=4
  Compare To Constant : simulink/Logic and Bit Operations/Compare To Constant  relop===  const=AEB_ObjectMovingState.Still  OutDataTypeStr=boolean
  Compare To Constant6 : simulink/Logic and Bit Operations/Compare To Constant  relop===  const=FALSE  OutDataTypeStr=boolean
  Constant : Constant  Value=P_AEB_Func_vehAEBfullObjMovingEscapeAborThres
  Constant1 : Constant  Value=P_AEB_Func_vehAEBfullObjStandEscapeAborThres
  Logical Operator1 : Logic  Operator=OR  AllPortsSameDT=off  OutDataTypeStr=boolean
  OverlapThesLookupTable : Lookup_n-D  NumberOfTableDimensions=1  BreakpointsForDimension1=P_AEB_Func_vehAEBfullOverlapAborThresX  BeginIndexSearchUsingPreviousIndexResult=on  Table=P_AEB_Func_vehAEBfullOverlapAborThresY  ExtrapMethod=Clip  InternalRulePriority=Speed  RndMeth=Simplest  InputSameDT=on
  Relational Operator : RelationalOperator  Operator=>  OutDataTypeStr=boolean  RndMeth=Simplest
  Relational Operator1 : RelationalOperator  Operator=>  OutDataTypeStr=boolean  RndMeth=Simplest
  Switch : Switch  Criteria=u2 ~= 0
  vehAEBfullSituationRelAborCondFulfilled : Outport
  wires:
  probEscape/1 -> Relational Operator1/1  "probEscape"
  Switch/1 -> Relational Operator1/2
  Constant/1 -> Switch/3
  Constant1/1 -> Switch/1
  Compare To Constant/1 -> Switch/2
  ObjectMovingState/1 -> Compare To Constant/1  "ObjectMovingState"
  Compare To Constant6/1 -> Logical Operator1/1  "OverlapCondFulfilled"
  Logical Operator1/1 -> vehAEBfullSituationRelAborCondFulfilled/1  "vehAEBfullSituationRelAborCondFulfilled"
  overlapRatio/1 -> Relational Operator/1  "overlapRatio"
  Relational Operator1/1 -> Logical Operator1/2  "probEscapeCondFulfilled"
  Relational Operator/1 -> Compare To Constant6/1  "OverlapCondFulfilled"
  OverlapThesLookupTable/1 -> Relational Operator/2
  dxFrontBumper/1 -> OverlapThesLookupTable/1

== system TosAeb/FcwAebJa_Step_sys/ALG_AEBSystem/ALG_AEBController/AEB_FuncCondCalcApp/LongFunctionCondCalc/vehAEBfullSuppAborCondCalc/vehAEBfullAborCondCalc/vehAEBfullVehRelAborCondCalc ==
  SteerWheelAngle : Inport
  SteerWheelSpd : Inport  Port=2
  AccrPedlPosnDiagc : Inport  Port=3
  vehStateCalcAEBfullAborFlg : Inport  Port=4
  DriverAborCondJudge : Subsystem
  vehAEBfullVehRelAborCondJudge : Subsystem
  vehAEBfullVehRelAborCondFulfilled : Outport
  wires:
  vehAEBfullVehRelAborCondJudge/1(vehAEBfullVehRelAborCondFulfilled) -> vehAEBfullVehRelAborCondFulfilled/1  "vehAEBfullVehRelAborCondFulfilled"
  vehStateCalcAEBfullAborFlg/1 -> vehAEBfullVehRelAborCondJudge/2(vehStateCalcAEBfullAborFlg)
  DriverAborCondJudge/1(driverAborCondFlg) -> vehAEBfullVehRelAborCondJudge/1(driverAborCondFlg)
  AccrPedlPosnDiagc/1 -> DriverAborCondJudge/3(AccrPedlPosnDiagc)
  SteerWheelSpd/1 -> DriverAborCondJudge/2(SteerWheelSpd)
  SteerWheelAngle/1 -> DriverAborCondJudge/1(SteerWheelAngle)

== system TosAeb/FcwAebJa_Step_sys/ALG_AEBSystem/ALG_AEBController/AEB_FuncCondCalcApp/LongFunctionCondCalc/vehAEBpartSuppAborCondCalc/vehAEBpartAborCondCalc/vehAEBpartAborCondFinalJudge ==
  vehAEBpartObjRelAborCondFulfilled : Inport
  vehAEBpartVehRelAborCondFulfilled : Inport  Port=2
  vehAEBpartSituationRelAborCondFulfilled : Inport  Port=3
  Compare To Constant : simulink/Logic and Bit Operations/Compare To Constant  relop===  const=TRUE  OutDataTypeStr=boolean
  Compare To Constant1 : simulink/Logic and Bit Operations/Compare To Constant  relop===  const=TRUE  OutDataTypeStr=boolean
  Compare To Constant2 : simulink/Logic and Bit Operations/Compare To Constant  relop===  const=TRUE  OutDataTypeStr=boolean
  Logical Operator : Logic  Operator=OR  Inputs=3  AllPortsSameDT=off  OutDataTypeStr=boolean
  vehAEBpartAborCondNoStandstillFulfilled : Outport
  vehAEBpartAborCondStandstillFulfilled : Outport  Port=2
  wires:
  Compare To Constant2/1 -> Logical Operator/2
  Compare To Constant1/1 -> Logical Operator/3, vehAEBpartAborCondStandstillFulfilled/1
  Compare To Constant/1 -> Logical Operator/1
  Logical Operator/1 -> vehAEBpartAborCondNoStandstillFulfilled/1
  vehAEBpartSituationRelAborCondFulfilled/1 -> Compare To Constant2/1
  vehAEBpartObjRelAborCondFulfilled/1 -> Compare To Constant/1
  vehAEBpartVehRelAborCondFulfilled/1 -> Compare To Constant1/1

== system TosAeb/FcwAebJa_Step_sys/ALG_AEBSystem/ALG_AEBController/AEB_FuncCondCalcApp/LongFunctionCondCalc/vehAEBpartSuppAborCondCalc/vehAEBpartAborCondCalc/vehAEBpartObjRelAborCondCalc ==
  ObjectID : Inport
  ObjectExistProb : Inport  Port=2
  ObjectIsObstacleProb : Inport  Port=3
  Compare To Constant4 : simulink/Logic and Bit Operations/Compare To Constant  relop===  const=CNobjInvalidID  OutDataTypeStr=boolean
  Compare To Constant6 : simulink/Logic and Bit Operations/Compare To Constant  relop=<  const=P_AEB_Func_vehAEBpartObjObstacleProbAborThres  OutDataTypeStr=boolean
  Compare To Constant7 : simulink/Logic and Bit Operations/Compare To Constant  relop=~=  const=CNobjInvalidID  OutDataTypeStr=boolean
  Logical Operator1 : Logic  Operator=OR  AllPortsSameDT=off  OutDataTypeStr=boolean
  Logical Operator3 : Logic  AllPortsSameDT=off  OutDataTypeStr=boolean
  Logical Operator5 : Logic  Operator=OR  AllPortsSameDT=off  OutDataTypeStr=boolean
  Product : Product
  Relational Operator : RelationalOperator  Operator=~=  OutDataTypeStr=boolean  RndMeth=Simplest
  Unit Delay : UnitDelay  SampleTime=-1  HasFrameUpgradeWarning=on
  vehAEBpartObjRelAborCondFulfilled : Outport
  wires:
  ObjectExistProb/1 -> Product/1
  Logical Operator5/1 -> vehAEBpartObjRelAborCondFulfilled/1  "vehAEBpartObjRelAborCondFulfilled"
  Product/1 -> Compare To Constant6/1
  Compare To Constant7/1 -> Logical Operator3/1
  ObjectIsObstacleProb/1 -> Product/2
  Logical Operator3/1 -> Logical Operator5/2  "objQualityAborCondFulfilled"
  Compare To Constant6/1 -> Logical Operator3/2
  ObjectID/1 -> Unit Delay/1, Compare To Constant7/1, Relational Operator/1, Compare To Constant4/1
  Unit Delay/1 -> Relational Operator/2
  Relational Operator/1 -> Logical Operator1/2
  Compare To Constant4/1 -> Logical Operator1/1
  Logical Operator1/1 -> Logical Operator5/1  "ObjIDAborCondFulfilled"
